simulink model "ofdm_txrx_supermimo"  (Simulink 7.1)
settings: Solver=VariableStepDiscrete  SolverMode=SingleTasking  StartTime=0.0  StopTime=simOnly_simLength  RelTol=1e-3
PostLoadFcn:
  | ofdm_rx_supermimo_init
InitFcn:
  | ofdm_rx_supermimo_init
StartFcn:
  | ofdm_rx_supermimo_init
values from neighbouring files (symbols this model uses):
  Action0_Config = 0* 31*2^18 + 0*2^24 + PHY_AUTORESPONSE_REQ_GOODHDR + PHY_AUTORESPONSE_REQ_GOODPKT + PHY_AUTORESPONSE_REQ_FLAGA    [ofdm_tx_supermimo_init.m]
  Action1_Config = 0* 62*2^18 + PHY_AUTORESPONSE_REQ_GOODHDR + PHY_AUTORESPONSE_REQ_GOODPKT    [ofdm_tx_supermimo_init.m]
  Action2_Config = 0    [ofdm_tx_supermimo_init.m]
  Action3_Config = 0    [ofdm_tx_supermimo_init.m]
  Action4_Config = 0    [ofdm_tx_supermimo_init.m]
  Action5_Config = 0    [ofdm_tx_supermimo_init.m]
  ActionID_AFTransmit = 31    [ofdm_tx_supermimo_init.m]
  ActionID_Disabled = 0    [ofdm_tx_supermimo_init.m]
  ActionID_SetFlagA = 62    [ofdm_tx_supermimo_init.m]
  ActionID_SetFlagB = 61    [ofdm_tx_supermimo_init.m]
  BER_RAM_init_size = 2048    [ofdm_tx_supermimo_init.m]
  CPLength = 16    [ofdm_tx_supermimo_init.m]
  longCorr_coef_i = [-4*real(LTS) 0]    [ofdm_rx_supermimo_init.m]
  longCorr_coef_q = [4*imag(LTS) 0]    [ofdm_rx_supermimo_init.m]
  Match0_Config = 0    [ofdm_tx_supermimo_init.m]
  Match1_Config = 0    [ofdm_tx_supermimo_init.m]
  Match2_Config = 0    [ofdm_tx_supermimo_init.m]
  Match3_Config = 0    [ofdm_tx_supermimo_init.m]
  Match4_Config = 0    [ofdm_tx_supermimo_init.m]
  Match5_Config = 0    [ofdm_tx_supermimo_init.m]
  Match6_Config = 0    [ofdm_tx_supermimo_init.m]
  Match7_Config = 0    [ofdm_tx_supermimo_init.m]
  max_CP_length = 16    [ofdm_tx_supermimo_init.m]
  max_num_baseRateSymbols = 31    [ofdm_tx_supermimo_init.m]
  max_num_subcarriers = 64    [ofdm_tx_supermimo_init.m]
  max_num_trainingSymbols = 15    [ofdm_tx_supermimo_init.m]
  max_numBytes = 16384    [ofdm_tx_supermimo_init.m]
  max_OFDM_symbols = 511    [ofdm_tx_supermimo_init.m]
  modConstellation_qam16 = .75*[1 3 -1 -3]./3    [ofdm_tx_supermimo_init.m]
  modConstellation_qam64 = 0.875*[3 1 5 7 -3 -1 -5 -7]./7    [ofdm_tx_supermimo_init.m]
  modConstellation_qpsk = [1 -1]./sqrt(2)    [ofdm_tx_supermimo_init.m]
  numBaseRateSymbols = ceil(numHeaderBytes / numBytes_BaseRateOFDMSymbol)    [ofdm_tx_supermimo_init.m]
  numSubcarriers = 64    [ofdm_tx_supermimo_init.m]
  numTrainingSymbols = 2    [ofdm_tx_supermimo_init.m]
  pilot_indicies = 7 + ( (64-21) * 2^8) + ( (64-7) * 2^16) + (21 * 2^24)    (= 356068103)    [ofdm_tx_supermimo_init.m]
  pilotValues = hex2dec('A57D') + (2^16 * hex2dec('5A82'))    [ofdm_tx_supermimo_init.m]
  pkt_crossCorr_thresh = 0.7    [ofdm_rx_supermimo_init.m]
  pkt_energy_thresh = 0    [ofdm_rx_supermimo_init.m]
  pktByteNums = numHeaderBytes +  				byteIndex_numPayloadBytes(1)*2^8 +  				byteIndex_numPayloadBytes(2)*2^16 +  				byteIndex_simpleDynModMasks * 2^24    [ofdm_rx_supermimo_init.m]
  PktDet_Delay = 58+32 +      Symbol_Timing_Offset*2^7 +      0*2^16 +      (117* 2^24)    (= 1962935642)    [ofdm_rx_supermimo_init.m]
  pktTiming_count_payloadStart = pktTiming_count_lsStart + pktTiming_ls_num*pktTiming_ls_length    [ofdm_rx_supermimo_init.m]
  preamble = preamble_scale*[shortsyms_10 longsyms_2]    [ofdm_tx_supermimo_init.m]
  preamble_ROM = preamble_I_ROM + preamble_Q_ROM*2^16    [ofdm_tx_supermimo_init.m]
  RAM_init_size = max_numBytes    (= 16384)    [ofdm_tx_supermimo_init.m]
  reg_AF_Tx_Blanking = (320+80) + ( (320+80+79)*2^16)    (= 31392144)    [ofdm_rx_supermimo_init.m]
  reg_AF_Tx_Scaling = 2944    [ofdm_rx_supermimo_init.m]
  reg_Interrupt_PktBuf_Control = reg_InterruptControl +  	1 * 2^16 +  	0 * 2^24    (= 65656)    [ofdm_rx_supermimo_init.m]
  reg_PilotCalcParams = (4 * 2^16) + (minPilotChanMag)    (= 262184.96)    [ofdm_rx_supermimo_init.m]
  regRx_chanEst_minMag = ((0.5)*2^15) + ((0.5 * 2^15)*2^16)    (= 1073758208)    [ofdm_rx_supermimo_init.m]
  regRx_coarseCalcCorrection = round((1.3e-3 - 1.2982e-3)*2^32)    [ofdm_rx_supermimo_init.m]
  regRx_pilotCalcCorrection = round(1.00115 * 2^31)    [ofdm_rx_supermimo_init.m]
  regRx_preCFOoptions = 0 * 2^0 +  	1 * 2^1 +  	0    (= 2)    [ofdm_rx_supermimo_init.m]
  rx_controlBits = 1 * 2  		 + 1 * 4  		 + 0 * 8  		 + 1 * 16 * rx_SISO_Mode  		 + 1 * 32  		 + 1 * 64  		 + 1 * 128  		 + 1 * 256  		 + 1 * 512  		 + 1 * 1024  		 + 1 * 4096  		 + 0 * 16384  		 + 0 * 32768  		 + 1 * 65536  		 + 0 * 131072  		 + 0 * 262144  		 + 1 * 524288  		 + 1 * 1048576 * tx_Alamouti_Mode  		 + 0 * 2097152           + 1 * 33554432  		 + 0 * 4194304  		 + 0 * 8388608  		 + 0 * 16777216  		 + 1 *  ...(+49 chars)    [ofdm_rx_supermimo_init.m]
  Rx_PktDet_LongCorr_Params = LongCorrCounterLoad + 0*2^8 + 0*2^16 + 255*2^24    (= 4278190325)    [ofdm_rx_supermimo_init.m]
  Rx_PktDet_LongCorrThresholds = 8000    [ofdm_rx_supermimo_init.m]
  rx_postEq_scaling = round(rxScaling*2^11) * (1 + 2^16)    [ofdm_rx_supermimo_init.m]
  RxDataScrambling_Seq = [40 198 78 63 82 173 102 245 48 111 172 115 147 230 216 93 72 65 62 2 205 242 122 90 128 83 105 97 73 10 5 252]    [ofdm_tx_supermimo_init.m]
  RxReg_FixedPktLen = 0    [ofdm_rx_supermimo_init.m]
  shortSymbol_time = shortSymbol_time(1:16).'    [ofdm_tx_supermimo_init.m]
  simOnly_numSamples = length(preamble)+( (numSubcarriers+CPLength)*(numTrainingSymbols + numBaseRateSymbols + numFullRateSymbols/2) )    [ofdm_tx_supermimo_init.m]
  train = [train train]    [ofdm_tx_supermimo_init.m]
  tx_controlBits = (antB_preambleShift * 2^4) + (2^1 * tx_Alamouti_Mode) +  	(2^0 * tx_SISO_Mode) + 4*0 + 8*1 + 0*256 + 512*0 + 1024*0 +  	0*hex2dec('1000') + 0*hex2dec('2000') + 0*hex2dec('4000') +  	1*hex2dec('8000') + 1*hex2dec('10000') + hex2dec('20000') +  	16*hex2dec('100000')    [ofdm_tx_supermimo_init.m]
  tx_delays = (50*2^0) + (0*2^8) + (30*2^12)    (= 122930)    [ofdm_tx_supermimo_init.m]
  tx_scaling = (3072) + (12288 * 2^16)    (= 805309440)    [ofdm_tx_supermimo_init.m]
  TxDataScrambling_Seq = [40 198 78 63 82 173 102 245 48 111 172 115 147 230 216 93 72 65 62 2 205 242 122 90 128 83 105 97 73 10 5 252]    [ofdm_tx_supermimo_init.m]
  txfilt_firpm = 0.95*txfilt_firpm./(max(txfilt_firpm))    [ofdm_tx_supermimo_init.m]
  txfilt_intfilt = [0 intfilt(4,4,.8)]    [ofdm_tx_supermimo_init.m]
  txReg_symbolCounts = (2^8 *numBaseRateSymbols ) + numTrainingSymbols    [ofdm_tx_supermimo_init.m]
  TxRx_FFTScaling = (3 * 2^0) +  	(2 * 2^2) +  	(1 * 2^4) +  	(1 * 2^6) +  	(1 * 2^8) +  	(0 * 2^10)    (= 347)    [ofdm_tx_supermimo_init.m]
undefined symbols (not defined in the file or in its folder): AntA, AntB, BaseRate, Down, gcbh, numBuffs, numBytes, Up, xlBlockLoadCallback, xlGetSimulinkPeriod, xlipgetpartsetting
libraries: xbsIndex_r4 x6035, simulink x4, dspsigops x4, commchan3 x2, dspsrcs4 x2, commrflib2 x2
(part 27 of 61)

== system ofdm_txrx_supermimo/OFDM Tx MIMO/Pre-Spin,  Filters & DACs/Filters/Interp Filt4 ==
  X : Inport  OutDataType=sfix(16)  OutScaling=2^0
  AddSub{1,3..4,7} (4 alike) : xbsIndex_r4/AddSub  mode=Addition  use_carryin=off  use_carryout=off  en=off  latency=0  precision=Full  arith_type=Unsigned  n_bits=16  bin_pt=14  quantization=Truncate  overflow=Wrap  dbl_ovrd=off  use_behavioral_HDL=off  pipelined=off  use_rpm=on  hw_selection=Fabric  block_type=addsub
      settings: block_version=10.1.3
  AddSub2 : xbsIndex_r4/AddSub  mode=Addition  use_carryin=off  use_carryout=off  en=off  latency=1  precision=Full  arith_type=Unsigned  n_bits=16  bin_pt=14  quantization=Truncate  overflow=Wrap  dbl_ovrd=off  use_behavioral_HDL=off  pipelined=off  use_rpm=on  hw_selection=Fabric  block_type=addsub
      settings: block_version=10.1.3
  AddSub5 : xbsIndex_r4/AddSub  mode=Addition  use_carryin=off  use_carryout=off  en=off  latency=1  precision=Full  arith_type=Unsigned  n_bits=16  bin_pt=14  quantization=Truncate  overflow=Wrap  dbl_ovrd=off  use_behavioral_HDL=off  pipelined=off  use_rpm=on  hw_selection=Fabric  block_type=addsub
      settings: block_version=10.1.3
  AddSub8 : xbsIndex_r4/AddSub  mode=Addition  use_carryin=off  use_carryout=off  en=off  latency=0  precision=User Defined  arith_type="Signed  (2's comp)"  n_bits=add_prec  bin_pt=add_bp  quantization=Truncate  overflow=Flag as error  dbl_ovrd=off  use_behavioral_HDL=off  pipelined=off  use_rpm=on  hw_selection=Fabric  block_type=addsub
      settings: block_version=10.1.3
  Counter : xbsIndex_r4/Counter  cnt_type=Free Running  cnt_to=Inf  operation=Up  start_count=1  cnt_by_val=1  arith_type=Unsigned  n_bits=2  bin_pt=0  load_pin=off  rst=off  en=off  explicit_period=on  period=data_samplePeriod / polyphaseBranches  dbl_ovrd=off  use_behavioral_HDL=off  use_rpm=off  implementation=Fabric  block_type=counter
  Delay{,1..6} (7 alike) : xbsIndex_r4/Delay  Orientation=down  en=off  latency=1  dbl_ovrd=off  reg_retiming=off  block_type=delay
  Mult{1..8} (8 alike) : xbsIndex_r4/Mult  precision=User Defined  arith_type="Signed  (2's comp)"  n_bits=mult_prec  bin_pt=mult_bp  quantization=Truncate  overflow=Flag as error  en=off  latency=multLatency  dbl_ovrd=off  use_behavioral_HDL=off  use_embedded=on  opt=Speed  optimum_pipeline=off  pipeline=on  use_rpm=on  placement_style=Triangular  block_type=mult
      settings: infoedit=Hardware notes: To use the internal pipeline stage of the dedicated multiplier you must select 'Pipeline for maximum performance'.  block_version=10.1.3
  ROM0 : xbsIndex_r4/ROM  depth=polyphaseBranches  initVector=h(1:subFilterLength)  distributed_mem=Distributed memory  rst=off  init_reg=0  en=off  latency=1  arith_type="Signed  (2's comp)"  n_bits=coeff_prec  bin_pt=coeff_bp  dbl_ovrd=off  optimize=Area  use_rpm=on  block_type=sprom
      settings: block_version=10.1.3
  ROM1 : xbsIndex_r4/ROM  depth=polyphaseBranches  initVector=h(1*subFilterLength+1:2*subFilterLength)  distributed_mem=Distributed memory  rst=off  init_reg=0  en=off  latency=1  arith_type="Signed  (2's comp)"  n_bits=coeff_prec  bin_pt=coeff_bp  dbl_ovrd=off  optimize=Area  use_rpm=on  block_type=sprom
      settings: block_version=10.1.3
  ROM2 : xbsIndex_r4/ROM  depth=polyphaseBranches  initVector=h(2*subFilterLength+1:3*subFilterLength)  distributed_mem=Distributed memory  rst=off  init_reg=0  en=off  latency=1  arith_type="Signed  (2's comp)"  n_bits=coeff_prec  bin_pt=coeff_bp  dbl_ovrd=off  optimize=Area  use_rpm=on  block_type=sprom
      settings: block_version=10.1.3
  ROM3 : xbsIndex_r4/ROM  depth=polyphaseBranches  initVector=h(3*subFilterLength+1:4*subFilterLength)  distributed_mem=Distributed memory  rst=off  init_reg=0  en=off  latency=1  arith_type="Signed  (2's comp)"  n_bits=coeff_prec  bin_pt=coeff_bp  dbl_ovrd=off  optimize=Area  use_rpm=on  block_type=sprom
      settings: block_version=10.1.3
  ROM4 : xbsIndex_r4/ROM  depth=polyphaseBranches  initVector=h(4*subFilterLength+1:5*subFilterLength)  distributed_mem=Distributed memory  rst=off  init_reg=0  en=off  latency=1  arith_type="Signed  (2's comp)"  n_bits=coeff_prec  bin_pt=coeff_bp  dbl_ovrd=off  optimize=Area  use_rpm=on  block_type=sprom
      settings: block_version=10.1.3
  ROM5 : xbsIndex_r4/ROM  depth=polyphaseBranches  initVector=h(5*subFilterLength+1:6*subFilterLength)  distributed_mem=Distributed memory  rst=off  init_reg=0  en=off  latency=1  arith_type="Signed  (2's comp)"  n_bits=coeff_prec  bin_pt=coeff_bp  dbl_ovrd=off  optimize=Area  use_rpm=on  block_type=sprom
      settings: block_version=10.1.3
  ROM6 : xbsIndex_r4/ROM  depth=polyphaseBranches  initVector=h(6*subFilterLength+1:7*subFilterLength)  distributed_mem=Distributed memory  rst=off  init_reg=0  en=off  latency=1  arith_type="Signed  (2's comp)"  n_bits=coeff_prec  bin_pt=coeff_bp  dbl_ovrd=off  optimize=Area  use_rpm=on  block_type=sprom
      settings: block_version=10.1.3
  ROM7 : xbsIndex_r4/ROM  depth=polyphaseBranches  initVector=h(7*subFilterLength+1:8*subFilterLength)  distributed_mem=Distributed memory  rst=off  init_reg=0  en=off  latency=1  arith_type="Signed  (2's comp)"  n_bits=coeff_prec  bin_pt=coeff_bp  dbl_ovrd=off  optimize=Area  use_rpm=on  block_type=sprom
      settings: block_version=10.1.3
  Up Sample{8..15} (8 alike) : xbsIndex_r4/Up Sample  sample_ratio=polyphaseBranches  copy_samples=on  dbl_ovrd=off  block_type=usamp
      settings: infoedit="Up samples input data.  Inserted values  can be zeros or copies of the most recent input sample.<P><P>Hardware notes: No hardware is needed if inserted values are copies of the input sample; otherwise, a mux and single bit flip-flop are used."  block_version=10.1.3
  Y : Outport  OutDataType=sfix(16)  OutScaling=2^0
  wires:
  AddSub8/1 -> Y/1
  Up Sample15/1 -> Mult5/1
  Up Sample14/1 -> Mult6/1
  Up Sample13/1 -> Mult4/1
  Up Sample12/1 -> Mult7/1
  Up Sample11/1 -> Mult2/1
  Up Sample10/1 -> Mult3/1
  Up Sample9/1 -> Mult1/1
  Up Sample8/1 -> Mult8/1
  AddSub5/1 -> AddSub8/2
  AddSub2/1 -> AddSub8/1
  Mult5/1 -> AddSub4/2
  Mult6/1 -> AddSub4/1
  Mult4/1 -> AddSub3/2
  Mult7/1 -> AddSub3/1
  AddSub4/1 -> AddSub5/2
  AddSub3/1 -> AddSub5/1
  AddSub1/1 -> AddSub2/2
  AddSub7/1 -> AddSub2/1
  Mult2/1 -> AddSub1/2
  Mult3/1 -> AddSub1/1
  Mult1/1 -> AddSub7/2
  Mult8/1 -> AddSub7/1
  Counter/1 -> ROM7/1, ROM6/1, ROM5/1, ROM4/1, ROM3/1, ROM2/1, ROM1/1, ROM0/1
  ROM7/1 -> Mult5/2
  ROM6/1 -> Mult6/2
  ROM5/1 -> Mult4/2
  ROM4/1 -> Mult7/2
  ROM3/1 -> Mult2/2
  ROM2/1 -> Mult3/2
  ROM1/1 -> Mult1/2
  ROM0/1 -> Mult8/2
  X/1 -> Delay/1, Up Sample8/1
  Delay6/1 -> Up Sample15/1
  Delay5/1 -> Delay6/1, Up Sample14/1
  Delay4/1 -> Delay5/1, Up Sample13/1
  Delay3/1 -> Delay4/1, Up Sample12/1
  Delay2/1 -> Delay3/1, Up Sample11/1
  Delay1/1 -> Delay2/1, Up Sample10/1
  Delay/1 -> Delay1/1, Up Sample9/1

== system ofdm_txrx_supermimo/OFDM Tx MIMO/Pre-Spin,  Filters & DACs/Filters/Interp Filt5 ==
  X : Inport  OutDataType=sfix(16)  OutScaling=2^0
  AddSub{1,3..4,7} (4 alike) : xbsIndex_r4/AddSub  mode=Addition  use_carryin=off  use_carryout=off  en=off  latency=0  precision=Full  arith_type=Unsigned  n_bits=16  bin_pt=14  quantization=Truncate  overflow=Wrap  dbl_ovrd=off  use_behavioral_HDL=off  pipelined=off  use_rpm=on  hw_selection=Fabric  block_type=addsub
      settings: block_version=10.1.3
  AddSub2 : xbsIndex_r4/AddSub  mode=Addition  use_carryin=off  use_carryout=off  en=off  latency=1  precision=Full  arith_type=Unsigned  n_bits=16  bin_pt=14  quantization=Truncate  overflow=Wrap  dbl_ovrd=off  use_behavioral_HDL=off  pipelined=off  use_rpm=on  hw_selection=Fabric  block_type=addsub
      settings: block_version=10.1.3
  AddSub5 : xbsIndex_r4/AddSub  mode=Addition  use_carryin=off  use_carryout=off  en=off  latency=1  precision=Full  arith_type=Unsigned  n_bits=16  bin_pt=14  quantization=Truncate  overflow=Wrap  dbl_ovrd=off  use_behavioral_HDL=off  pipelined=off  use_rpm=on  hw_selection=Fabric  block_type=addsub
      settings: block_version=10.1.3
  AddSub8 : xbsIndex_r4/AddSub  mode=Addition  use_carryin=off  use_carryout=off  en=off  latency=0  precision=User Defined  arith_type="Signed  (2's comp)"  n_bits=add_prec  bin_pt=add_bp  quantization=Truncate  overflow=Flag as error  dbl_ovrd=off  use_behavioral_HDL=off  pipelined=off  use_rpm=on  hw_selection=Fabric  block_type=addsub
      settings: block_version=10.1.3
  Counter : xbsIndex_r4/Counter  cnt_type=Free Running  cnt_to=Inf  operation=Up  start_count=1  cnt_by_val=1  arith_type=Unsigned  n_bits=2  bin_pt=0  load_pin=off  rst=off  en=off  explicit_period=on  period=data_samplePeriod / polyphaseBranches  dbl_ovrd=off  use_behavioral_HDL=off  use_rpm=off  implementation=Fabric  block_type=counter
  Delay{,1..6} (7 alike) : xbsIndex_r4/Delay  Orientation=down  en=off  latency=1  dbl_ovrd=off  reg_retiming=off  block_type=delay
  Mult{1..8} (8 alike) : xbsIndex_r4/Mult  precision=User Defined  arith_type="Signed  (2's comp)"  n_bits=mult_prec  bin_pt=mult_bp  quantization=Truncate  overflow=Flag as error  en=off  latency=multLatency  dbl_ovrd=off  use_behavioral_HDL=off  use_embedded=on  opt=Speed  optimum_pipeline=off  pipeline=on  use_rpm=on  placement_style=Triangular  block_type=mult
      settings: infoedit=Hardware notes: To use the internal pipeline stage of the dedicated multiplier you must select 'Pipeline for maximum performance'.  block_version=10.1.3
  ROM0 : xbsIndex_r4/ROM  depth=polyphaseBranches  initVector=h(1:subFilterLength)  distributed_mem=Distributed memory  rst=off  init_reg=0  en=off  latency=1  arith_type="Signed  (2's comp)"  n_bits=coeff_prec  bin_pt=coeff_bp  dbl_ovrd=off  optimize=Area  use_rpm=on  block_type=sprom
      settings: block_version=10.1.3
  ROM1 : xbsIndex_r4/ROM  depth=polyphaseBranches  initVector=h(1*subFilterLength+1:2*subFilterLength)  distributed_mem=Distributed memory  rst=off  init_reg=0  en=off  latency=1  arith_type="Signed  (2's comp)"  n_bits=coeff_prec  bin_pt=coeff_bp  dbl_ovrd=off  optimize=Area  use_rpm=on  block_type=sprom
      settings: block_version=10.1.3
  ROM2 : xbsIndex_r4/ROM  depth=polyphaseBranches  initVector=h(2*subFilterLength+1:3*subFilterLength)  distributed_mem=Distributed memory  rst=off  init_reg=0  en=off  latency=1  arith_type="Signed  (2's comp)"  n_bits=coeff_prec  bin_pt=coeff_bp  dbl_ovrd=off  optimize=Area  use_rpm=on  block_type=sprom
      settings: block_version=10.1.3
  ROM3 : xbsIndex_r4/ROM  depth=polyphaseBranches  initVector=h(3*subFilterLength+1:4*subFilterLength)  distributed_mem=Distributed memory  rst=off  init_reg=0  en=off  latency=1  arith_type="Signed  (2's comp)"  n_bits=coeff_prec  bin_pt=coeff_bp  dbl_ovrd=off  optimize=Area  use_rpm=on  block_type=sprom
      settings: block_version=10.1.3
  ROM4 : xbsIndex_r4/ROM  depth=polyphaseBranches  initVector=h(4*subFilterLength+1:5*subFilterLength)  distributed_mem=Distributed memory  rst=off  init_reg=0  en=off  latency=1  arith_type="Signed  (2's comp)"  n_bits=coeff_prec  bin_pt=coeff_bp  dbl_ovrd=off  optimize=Area  use_rpm=on  block_type=sprom
      settings: block_version=10.1.3
  ROM5 : xbsIndex_r4/ROM  depth=polyphaseBranches  initVector=h(5*subFilterLength+1:6*subFilterLength)  distributed_mem=Distributed memory  rst=off  init_reg=0  en=off  latency=1  arith_type="Signed  (2's comp)"  n_bits=coeff_prec  bin_pt=coeff_bp  dbl_ovrd=off  optimize=Area  use_rpm=on  block_type=sprom
      settings: block_version=10.1.3
  ROM6 : xbsIndex_r4/ROM  depth=polyphaseBranches  initVector=h(6*subFilterLength+1:7*subFilterLength)  distributed_mem=Distributed memory  rst=off  init_reg=0  en=off  latency=1  arith_type="Signed  (2's comp)"  n_bits=coeff_prec  bin_pt=coeff_bp  dbl_ovrd=off  optimize=Area  use_rpm=on  block_type=sprom
      settings: block_version=10.1.3
  ROM7 : xbsIndex_r4/ROM  depth=polyphaseBranches  initVector=h(7*subFilterLength+1:8*subFilterLength)  distributed_mem=Distributed memory  rst=off  init_reg=0  en=off  latency=1  arith_type="Signed  (2's comp)"  n_bits=coeff_prec  bin_pt=coeff_bp  dbl_ovrd=off  optimize=Area  use_rpm=on  block_type=sprom
      settings: block_version=10.1.3
  Up Sample{8..15} (8 alike) : xbsIndex_r4/Up Sample  sample_ratio=polyphaseBranches  copy_samples=on  dbl_ovrd=off  block_type=usamp
      settings: infoedit="Up samples input data.  Inserted values  can be zeros or copies of the most recent input sample.<P><P>Hardware notes: No hardware is needed if inserted values are copies of the input sample; otherwise, a mux and single bit flip-flop are used."  block_version=10.1.3
  Y : Outport  OutDataType=sfix(16)  OutScaling=2^0
  wires:
  Delay/1 -> Up Sample9/1, Delay1/1
  Delay1/1 -> Up Sample10/1, Delay2/1
  Delay2/1 -> Up Sample11/1, Delay3/1
  Delay3/1 -> Up Sample12/1, Delay4/1
  Delay4/1 -> Up Sample13/1, Delay5/1
  Delay5/1 -> Up Sample14/1, Delay6/1
  Delay6/1 -> Up Sample15/1
  X/1 -> Up Sample8/1, Delay/1
  ROM0/1 -> Mult8/2
  ROM1/1 -> Mult1/2
  ROM2/1 -> Mult3/2
  ROM3/1 -> Mult2/2
  ROM4/1 -> Mult7/2
  ROM5/1 -> Mult4/2
  ROM6/1 -> Mult6/2
  ROM7/1 -> Mult5/2
  Counter/1 -> ROM0/1, ROM1/1, ROM2/1, ROM3/1, ROM4/1, ROM5/1, ROM6/1, ROM7/1
  Mult8/1 -> AddSub7/1
  Mult1/1 -> AddSub7/2
  Mult3/1 -> AddSub1/1
  Mult2/1 -> AddSub1/2
  AddSub7/1 -> AddSub2/1
  AddSub1/1 -> AddSub2/2
  AddSub3/1 -> AddSub5/1
  AddSub4/1 -> AddSub5/2
  Mult7/1 -> AddSub3/1
  Mult4/1 -> AddSub3/2
  Mult6/1 -> AddSub4/1
  Mult5/1 -> AddSub4/2
  AddSub2/1 -> AddSub8/1
  AddSub5/1 -> AddSub8/2
  Up Sample8/1 -> Mult8/1
  Up Sample9/1 -> Mult1/1
  Up Sample10/1 -> Mult3/1
  Up Sample11/1 -> Mult2/1
  Up Sample12/1 -> Mult7/1
  Up Sample13/1 -> Mult4/1
  Up Sample14/1 -> Mult6/1
  Up Sample15/1 -> Mult5/1
  AddSub8/1 -> Y/1

== system ofdm_txrx_supermimo/OFDM Tx MIMO/Training_Data/Ant Selector/Oscillator ==
  rst : Inport  OutDataType=sfix(16)  OutScaling=2^0
  en : Inport  Port=2  OutDataType=sfix(16)  OutScaling=2^0
  Assert : xbsIndex_r4/Assert  Orientation=left  assert_type=on  type_source=Explicitly  arith_type=Boolean  n_bits=16  bin_pt=14  assert_rate=on  rate_source=Explicitly  period=2  output_port=on  block_type=assert
      settings: infoedit=Asserts a user-defined sample rate and/or type on a signal.<P><P>Hardware notes: In hardware this block costs nothing.  block_version=VER_STRING_GOES_HERE
  Inverter1 : xbsIndex_r4/Inverter  Orientation=left  en=off  latency=0  dbl_ovrd=off  block_type=inv
      settings: block_version=VER_STRING_GOES_HERE
  Register : xbsIndex_r4/Register  init=0  rst=on  en=on  dbl_ovrd=off  block_type=register
      settings: block_version=VER_STRING_GOES_HERE
  Q : Outport  OutDataType=sfix(16)  OutScaling=2^0
  wires:
  en/1 -> Register/3
  Inverter1/1 -> Register/1
  Assert/1 -> Inverter1/1
  Register/1 -> Assert/1, Q/1
  rst/1 -> Register/2

== system ofdm_txrx_supermimo/OFDM Tx MIMO/Training_Data/Ant Selector/negedge ==
  D : Inport  OutDataType=sfix(16)  OutScaling=2^0
  Delay : xbsIndex_r4/Delay  en=off  latency=1  dbl_ovrd=off  reg_retiming=on  block_type=delay
      settings: infoedit=Hardware notes: A delay line is a chain, each link of which is an SRL16 followed by a flip-flop. If register retiming is enabled, the delay line is a chain of flip-flops.  block_version=VER_STRING_GOES_HERE
  Inverter1 : xbsIndex_r4/Inverter  en=off  latency=0  dbl_ovrd=off  block_type=inv
      settings: block_version=VER_STRING_GOES_HERE
  Logical3 : xbsIndex_r4/Logical  logical_function=AND  inputs=2  en=off  latency=0  precision=Full  arith_type=Unsigned  n_bits=16  bin_pt=0  align_bp=on  dbl_ovrd=off  block_type=logical
      settings: block_version=VER_STRING_GOES_HERE
  Q : Outport  OutDataType=sfix(16)  OutScaling=2^0
  wires:
  Inverter1/1 -> Logical3/1
  D/1 -> Inverter1/1, Delay/1
  Delay/1 -> Logical3/2
  Logical3/1 -> Q/1

== system ofdm_txrx_supermimo/OFDM Tx MIMO/Training_Data/FlexibleMod/PktBuffer_CRC1 ==
  Rst : Inport  OutDataType=sfix(16)  OutScaling=2^0
  DataOut_Addr Vin : Inport  Port=2  OutDataType=sfix(16)  OutScaling=2^0
  DataOut_Addr : Inport  Port=3  OutDataType=sfix(16)  OutScaling=2^0
  Assert : xbsIndex_r4/Assert  Orientation=left  assert_type=off  type_source=Explicitly  arith_type="Signed  (2's comp)"  n_bits=16  bin_pt=14  assert_rate=on  rate_source=Explicitly  period=2  output_port=on  block_type=assert
      settings: infoedit=Asserts a user-defined sample rate and/or type on a signal.<P><P>Hardware notes: In hardware this block costs nothing.  block_version=8.2.01
  Byte Selector : Subsystem
  CRC Length Calc1 : Subsystem
  CRC16 Calc : Subsystem
  CRC32 Calc : Subsystem
  Gateway Out1 : xbsIndex_r4/Gateway Out  hdl_port=off  timing_constraint=None  locs_specified=off  LOCs={}  block_type=gatewayout
      settings: infoedit="Gateway out block.  Converts Xilinx fixed point inputs into ouputs of type Simulink integer, double, or fixed point.<P><P>Hardware notes:  In hardware these blocks become top level output ports or are discarded, depending on how they are configured."  block_version=10.1.3
  Gateway Out4 : xbsIndex_r4/Gateway Out  hdl_port=off  timing_constraint=None  locs_specified=off  LOCs={}  block_type=gatewayout
      settings: infoedit="Gateway out block.  Converts Xilinx fixed point inputs into ouputs of type Simulink integer, double, or fixed point.<P><P>Hardware notes:  In hardware these blocks become top level output ports or are discarded, depending on how they are configured."  block_version=10.1.3
  Header Decoding1 : Subsystem
  Logical8 : xbsIndex_r4/Logical  logical_function=OR  inputs=2  en=off  latency=0  precision=Full  arith_type=Unsigned  n_bits=8  bin_pt=2  align_bp=on  dbl_ovrd=off  block_type=logical
      settings: xl_area=[0, 0, 0, 0, 0, 0, 0]  block_version=VER_STRING_GOES_HERE
  Mux : xbsIndex_r4/Mux  inputs=2  en=off  latency=0  precision=Full  arith_type="Signed  (2's comp)"  n_bits=8  bin_pt=2  quantization=Truncate  overflow=Wrap  dbl_ovrd=off  block_type=mux
      settings: xl_area=[0, 0, 0, 0, 0, 0, 0]  block_version=VER_STRING_GOES_HERE
  Packet Buffer : Subsystem
  Register{1,3..6} (5 alike) : xbsIndex_r4/Register  init=0  rst=off  en=off  dbl_ovrd=off  block_type=register
      settings: xl_area=[2 4 0 0 0 0 0]  block_version=VER_STRING_GOES_HERE
  Register2 : xbsIndex_r4/Register  Orientation=up  init=0  rst=off  en=off  dbl_ovrd=off  block_type=register
      settings: xl_area=[2 4 0 0 0 0 0]  block_version=VER_STRING_GOES_HERE
  Scrambling : Subsystem
  Tx Bytes : Scope  NumInputPorts=2
      settings: TimeRange=7000  YMin=0~0  YMax=3~4e+009
  NumBytes : Outport  OutDataType=sfix(16)  OutScaling=2^0
  FullRateMasks : Outport  Port=2  OutDataType=sfix(16)  OutScaling=2^0
  Dout : Outport  Port=3  OutDataType=sfix(16)  OutScaling=2^0
  wires:
  Header Decoding1/1(numBytes) -> NumBytes/1, CRC Length Calc1/1(NumBytes)
  Header Decoding1/2(modMask) -> FullRateMasks/1
  DataOut_Addr/1 -> Packet Buffer/1(TxByteAddr), Register2/1, Register4/1
  Scrambling/1(Dout) -> Dout/1
  Mux/1 -> Scrambling/1(Din), Assert/1, Gateway Out4/1
  Packet Buffer/1(ByteOut) -> Mux/2, Header Decoding1/2(TxByte)
  DataOut_Addr Vin/1 -> Register3/1
  Rst/1 -> Register1/1
  CRC Length Calc1/3(CRC_ByteSel) -> Byte Selector/2(ByteSel)
  Byte Selector/1(1 Byte Out) -> Mux/3
  CRC Length Calc1/4(CRC32_Capt) -> CRC32 Calc/4(Capt)
  Assert/1 -> CRC16 Calc/1(ByteIn), CRC32 Calc/1(ByteIn)
  Register3/1 -> Header Decoding1/3(TxByteAddrVin), CRC Length Calc1/3(Vin), CRC32 Calc/2(Vin), CRC16 Calc/2(Vin)
  Register1/1 -> CRC32 Calc/3(Rst), Header Decoding1/4(Rst), CRC16 Calc/3(Rst)
  Register2/1 -> Header Decoding1/1(TxByteAddr), Register6/1, Scrambling/2(Addr)
  CRC32 Calc/1(32-bit CRC Capt) -> Byte Selector/3(CRC32)
  CRC Length Calc1/5(CRC16_Capt) -> CRC16 Calc/4(Capt)
  CRC Length Calc1/1(CRC32_En) -> Logical8/1, Byte Selector/1(CRC32_en)
  CRC Length Calc1/2(CRC16_En) -> Logical8/2
  Logical8/1 -> Mux/1
  CRC16 Calc/1(16-bit CRC Capt) -> Byte Selector/4(CRC16)
  Gateway Out4/1 -> Tx Bytes/1
  Gateway Out1/1 -> Tx Bytes/2
  Register4/1 -> Register5/1
  Register5/1 -> Gateway Out1/1
  Register6/1 -> CRC Length Calc1/2(ByteAddr)

== system ofdm_txrx_supermimo/OFDM Tx MIMO/Training_Data/FlexibleMod/QAM ==
  note: Mod-masks are interpreted as literal bits/symbol value. Only
      | BPSK/QPSK/16QAM/64QAM are implemented (1/2/4/6 b/sym),
      | but other schemes could be added in this block to fill the unused
      | mod mask values.
  Mod Bits : Inport  OutDataType=sfix(16)  OutScaling=2^0
  Mod Sel : Inport  Port=2  OutDataType=sfix(16)  OutScaling=2^0
  16QAM Mapper : xbsIndex_r4/ROM  depth=max(size(modConstellation_qam16))  initVector=modConstellation_qam16  distributed_mem=Distributed memory  rst=off  init_reg=0  en=off  latency=1  arith_type="Signed  (2's comp)"  n_bits=modConstellation_prec  bin_pt=modConstellation_bp  dbl_ovrd=off  optimize=Area  use_rpm=off  block_type=sprom
      settings: xl_area=[4 8 0 8 0 0 0]  block_version=VER_STRING_GOES_HERE
  16QAM Mapper1 : xbsIndex_r4/ROM  depth=max(size(modConstellation_qam16))  initVector=modConstellation_qam16  distributed_mem=Distributed memory  rst=off  init_reg=0  en=off  latency=1  arith_type="Signed  (2's comp)"  n_bits=modConstellation_prec  bin_pt=modConstellation_bp  dbl_ovrd=off  optimize=Area  use_rpm=off  block_type=sprom
      settings: xl_area=[4 8 0 8 0 0 0]  block_version=VER_STRING_GOES_HERE
  1MSB : xbsIndex_r4/Slice  nbits=1  boolean_output=off  mode=Upper Bit Location + Width  bit1=0  base1=MSB of Input  bit0=0  base0=LSB of Input  dbl_ovrd=off  block_type=slice
      settings: infoedit="Extracts a given range of bits from each input sample and presents it at the output.  The output type is ordinarily unsigned with binary point at zero, but can be Boolean when the slice is one bit wide.<P><P>Hardware notes: In hardware this block costs nothing."  block_version=VER_STRING_GOES_HERE
  1MSB-1 : xbsIndex_r4/Slice  nbits=1  boolean_output=off  mode=Upper Bit Location + Width  bit1=-1  base1=MSB of Input  bit0=0  base0=LSB of Input  dbl_ovrd=off  block_type=slice
      settings: infoedit="Extracts a given range of bits from each input sample and presents it at the output.  The output type is ordinarily unsigned with binary point at zero, but can be Boolean when the slice is one bit wide.<P><P>Hardware notes: In hardware this block costs nothing."  block_version=VER_STRING_GOES_HERE
  2MSB : xbsIndex_r4/Slice  nbits=2  boolean_output=off  mode=Upper Bit Location + Width  bit1=0  base1=MSB of Input  bit0=0  base0=LSB of Input  dbl_ovrd=off  block_type=slice
      settings: infoedit="Extracts a given range of bits from each input sample and presents it at the output.  The output type is ordinarily unsigned with binary point at zero, but can be Boolean when the slice is one bit wide.<P><P>Hardware notes: In hardware this block costs nothing."  block_version=VER_STRING_GOES_HERE
  2MSB-2 : xbsIndex_r4/Slice  nbits=2  boolean_output=off  mode=Upper Bit Location + Width  bit1=-2  base1=MSB of Input  bit0=0  base0=LSB of Input  dbl_ovrd=off  block_type=slice
      settings: infoedit="Extracts a given range of bits from each input sample and presents it at the output.  The output type is ordinarily unsigned with binary point at zero, but can be Boolean when the slice is one bit wide.<P><P>Hardware notes: In hardware this block costs nothing."  block_version=VER_STRING_GOES_HERE
  3LSB : xbsIndex_r4/Slice  nbits=3  boolean_output=off  mode=Lower Bit Location + Width  bit1=0  base1=MSB of Input  bit0=0  base0=LSB of Input  dbl_ovrd=off  block_type=slice
      settings: infoedit="Extracts a given range of bits from each input sample and presents it at the output.  The output type is ordinarily unsigned with binary point at zero, but can be Boolean when the slice is one bit wide.<P><P>Hardware notes: In hardware this block costs nothing."  block_version=VER_STRING_GOES_HERE
  3MS-3 : xbsIndex_r4/Slice  nbits=3  boolean_output=off  mode=Upper Bit Location + Width  bit1=-3  base1=MSB of Input  bit0=0  base0=LSB of Input  dbl_ovrd=off  block_type=slice
      settings: infoedit="Extracts a given range of bits from each input sample and presents it at the output.  The output type is ordinarily unsigned with binary point at zero, but can be Boolean when the slice is one bit wide.<P><P>Hardware notes: In hardware this block costs nothing."  block_version=VER_STRING_GOES_HERE
  3MSB : xbsIndex_r4/Slice  nbits=3  boolean_output=off  mode=Upper Bit Location + Width  bit1=0  base1=MSB of Input  bit0=0  base0=LSB of Input  dbl_ovrd=off  block_type=slice
      settings: infoedit="Extracts a given range of bits from each input sample and presents it at the output.  The output type is ordinarily unsigned with binary point at zero, but can be Boolean when the slice is one bit wide.<P><P>Hardware notes: In hardware this block costs nothing."  block_version=VER_STRING_GOES_HERE
  64QAM Mapper : xbsIndex_r4/ROM  depth=max(size(modConstellation_qam64))  initVector=modConstellation_qam64  distributed_mem=Distributed memory  rst=off  init_reg=0  en=off  latency=1  arith_type="Signed  (2's comp)"  n_bits=modConstellation_prec  bin_pt=modConstellation_bp  dbl_ovrd=off  optimize=Area  use_rpm=off  block_type=sprom
      settings: xl_area=[4 8 0 8 0 0 0]  block_version=VER_STRING_GOES_HERE
  64QAM Mapper1 : xbsIndex_r4/ROM  depth=max(size(modConstellation_qam64))  initVector=modConstellation_qam64  distributed_mem=Distributed memory  rst=off  init_reg=0  en=off  latency=1  arith_type="Signed  (2's comp)"  n_bits=modConstellation_prec  bin_pt=modConstellation_bp  dbl_ovrd=off  optimize=Area  use_rpm=off  block_type=sprom
      settings: xl_area=[4 8 0 8 0 0 0]  block_version=VER_STRING_GOES_HERE
  Constant7 : xbsIndex_r4/Constant  arith_type=Signed (2's comp)  const=0  n_bits=1  bin_pt=0  explicit_period=off  period=1  equ=P=C  opselect=C  inp2=PCIN>>17  opr=+  inp1=P  carry=CIN  dbl_ovrd=off  block_type=constant
      settings: block_version=VER_STRING_GOES_HERE
  Delay : xbsIndex_r4/Delay  en=off  latency=1  dbl_ovrd=off  reg_retiming=off  block_type=delay
      settings: infoedit=Hardware notes: A delay line is a chain, each link of which is an SRL16 followed by a flip-flop. If register retiming is enabled, the delay line is a chain of flip-flops.  xl_area=[2 4 0 0 0 0 0]  block_version=VER_STRING_GOES_HERE
  Mux1 : xbsIndex_r4/Mux  inputs=7  en=off  latency=0  precision=Full  arith_type="Signed  (2's comp)"  n_bits=8  bin_pt=2  quantization=Truncate  overflow=Wrap  dbl_ovrd=off  block_type=mux
      settings: xl_area=[36 0 0 72 0 0 0]  block_version=VER_STRING_GOES_HERE
  Mux2 : xbsIndex_r4/Mux  inputs=7  en=off  latency=0  precision=Full  arith_type="Signed  (2's comp)"  n_bits=8  bin_pt=2  quantization=Truncate  overflow=Wrap  dbl_ovrd=off  block_type=mux
      settings: xl_area=[36 0 0 72 0 0 0]  block_version=VER_STRING_GOES_HERE
  QPSK Map : Subsystem
  QPSK Map1 : Subsystem
  I : Outport  OutDataType=sfix(16)  OutScaling=2^0
  Q : Outport  Port=2  OutDataType=sfix(16)  OutScaling=2^0
  wires:
  1MSB-1/1 -> QPSK Map1/1(Bit)
  1MSB/1 -> QPSK Map/1(Bit)
  2MSB-2/1 -> 16QAM Mapper1/1
  2MSB/1 -> 16QAM Mapper/1
  Mod Bits/1 -> 1MSB/1, 2MSB/1, 3MSB/1, 2MSB-2/1, 3MS-3/1, 1MSB-1/1
  Mux2/1 -> I/1
  Mux1/1 -> Q/1
  3MS-3/1 -> 64QAM Mapper1/1
  3MSB/1 -> 64QAM Mapper/1
  QPSK Map1/1(Sym) -> Mux1/4
  16QAM Mapper1/1 -> Mux1/6
  64QAM Mapper1/1 -> Mux1/8
  Mod Sel/1 -> 3LSB/1
  Delay/1 -> Mux2/1, Mux1/1
  Constant7/1 -> Mux2/2, Mux2/5, Mux2/7, Mux1/3, Mux1/5, Mux1/7, Mux1/2
  QPSK Map/1(Sym) -> Mux2/3, Mux2/4
  16QAM Mapper/1 -> Mux2/6
  64QAM Mapper/1 -> Mux2/8
  3LSB/1 -> Delay/1

== system ofdm_txrx_supermimo/OFDM Tx MIMO/Training_Data/FlexibleMod/S-R Latch1 ==
  R : Inport  OutDataType=sfix(16)  OutScaling=2^0
  S : Inport  Port=2  OutDataType=sfix(16)  OutScaling=2^0
  Constant3 : xbsIndex_r4/Constant  arith_type=Boolean  const=1  n_bits=1  bin_pt=0  explicit_period=off  period=1  equ=P=C  opselect=C  inp2=PCIN>>17  opr=+  inp1=P  carry=CIN  dbl_ovrd=off  block_type=constant
      settings: block_version=VER_STRING_GOES_HERE
  Register : xbsIndex_r4/Register  init=0  rst=on  en=on  dbl_ovrd=off  block_type=register
      settings: xl_area=[0, 0, 0, 0, 0, 0, 0]  block_version=VER_STRING_GOES_HERE
  Q : Outport  OutDataType=sfix(16)  OutScaling=2^0
  wires:
  Constant3/1 -> Register/1
  R/1 -> Register/2
  Register/1 -> Q/1
  S/1 -> Register/3

== system ofdm_txrx_supermimo/OFDM Tx MIMO/Training_Data/FlexibleMod/SR Latch ==
  R : Inport  OutDataType=sfix(16)  OutScaling=2^0
  S : Inport  Port=2  OutDataType=sfix(16)  OutScaling=2^0
  Constant2 : xbsIndex_r4/Constant  arith_type=Boolean  const=1  n_bits=1  bin_pt=0  explicit_period=off  period=1  equ=P=C  opselect=C  inp2=PCIN>>17  opr=+  inp1=P  carry=CIN  dbl_ovrd=off  block_type=constant
      settings: block_version=VER_STRING_GOES_HERE
  Convert : xbsIndex_r4/Convert  arith_type=Boolean  n_bits=16  bin_pt=14  quantization=Truncate  overflow=Wrap  latency=0  dbl_ovrd=off  pipeline=off  block_type=convert
      settings: block_version=8.2.01
  Convert1 : xbsIndex_r4/Convert  arith_type=Boolean  n_bits=16  bin_pt=14  quantization=Truncate  overflow=Wrap  latency=0  dbl_ovrd=off  pipeline=off  block_type=convert
      settings: block_version=8.2.01
  crc_accum2 : xbsIndex_r4/Register  init=0  rst=on  en=on  dbl_ovrd=off  block_type=register
      settings: xl_area=[0, 0, 0, 0, 0, 0, 0]  block_version=VER_STRING_GOES_HERE
  Q : Outport  OutDataType=sfix(16)  OutScaling=2^0
  wires:
  Constant2/1 -> crc_accum2/1
  R/1 -> Convert/1
  S/1 -> Convert1/1
  crc_accum2/1 -> Q/1
  Convert/1 -> crc_accum2/2
  Convert1/1 -> crc_accum2/3

== system ofdm_txrx_supermimo/OFDM Tx MIMO/Training_Data/FlexibleMod/control ==
  note: This input, sub sync, gives the user
      |  the ability to reset the subcarrier
      | counter so that the user can
      | control what subcarrier the system starts
      | putting data on.
  note: This input, ready, is a bit that
      | is asserted when the user
      | is ready for the system to run,
      | i.e. the user has loaded information
      |  into the memory and is ready to
      | handle the output.
  note: This input, length, is the length
      | of the packet loaded in memory.
  note: This output puts out a pulse when the entire
      | system is done taking the bytes from the memory.
  note: This output, mod, is the desired modulation
      | type for a given group of bits. The values of this
      | output is designated by the values in
      |  subcarrier_QAM_Values in the init file
  note: This ouput, index, provides the index for
      | the location in the two registersos  so that the registers
      | can know when to load and the slicer can know
      | which bits to slice off of the 16-bit output of data_buffer
  note: This subsystem acts as the control unit for the rest of the system.
      | It uses a state system to control the system with three states, on, resetting, and off.
      | The number of subcarriers should be a power of two.
  FullRateMasks : Inport  OutDataType=sfix(16)  OutScaling=2^0
  length : Inport  Port=2  OutDataType=sfix(16)  OutScaling=2^0
  ready : Inport  Port=3  OutDataType=sfix(16)  OutScaling=2^0
  ifft_index : Inport  Port=4  OutDataType=sfix(16)  OutScaling=2^0
  BaseRate_En : Inport  Port=5  OutDataType=sfix(16)  OutScaling=2^0
  valid : Inport  Port=6  OutDataType=sfix(16)  OutScaling=2^0
  ant_sel : Inport  Port=7  OutDataType=sfix(16)  OutScaling=2^0
  Accumulator : xbsIndex_r4/Accumulator  operation=Add  n_bits=1  overflow=Saturate  scale=1  rst=on  hasbypass=off  en=off  dbl_ovrd=off  use_behavioral_HDL=on  implementation=Fabric  block_type=accum
      settings: infoedit="Adder or subtractor-based accumulator.   Output type and binary point position match the input.<P><P>Hardware notes: When \"Reinitialize with input 'b' on reset\" is selected, the accumulator is forced to run at the system rate even if the input 'b' is running at a slower rate."  xl_area=[0, 0, 0, 0, 0, 0, 0]  block_version=VER_STRING_GOES_HERE
  Accumulator2 : xbsIndex_r4/Accumulator  operation=Add  n_bits=14  overflow=Wrap  scale=1  rst=on  hasbypass=off  en=on  dbl_ovrd=off  use_behavioral_HDL=on  implementation=Fabric  block_type=accum
      settings: infoedit="Adder or subtractor-based accumulator.   Output type and binary point position match the input.<P><P>Hardware notes: When \"Reinitialize with input 'b' on reset\" is selected, the accumulator is forced to run at the system rate even if the input 'b' is running at a slower rate."  xl_area=[7 14 0 14 0 0 0]  block_version=VER_STRING_GOES_HERE
  AddSub1 : xbsIndex_r4/AddSub  Orientation=left  mode=Addition  use_carryin=off  use_carryout=off  en=off  latency=0  precision=Full  arith_type=Unsigned  n_bits=8  bin_pt=2  quantization=Truncate  overflow=Wrap  dbl_ovrd=off  use_behavioral_HDL=on  pipelined=off  use_rpm=on  hw_selection=Fabric  block_type=addsub
      settings: xl_area=[8 0 0 14 0 0 0]  block_version=VER_STRING_GOES_HERE
  Assert : xbsIndex_r4/Assert  Orientation=left  assert_type=off  type_source=Explicitly  arith_type="Signed  (2's comp)"  n_bits=16  bin_pt=14  assert_rate=on  rate_source=Explicitly  period=2  output_port=on  block_type=assert
      settings: infoedit=Asserts a user-defined sample rate and/or type on a signal.<P><P>Hardware notes: In hardware this block costs nothing.  block_version=VER_STRING_GOES_HERE
  Concat : xbsIndex_r4/Concat  num_inputs=3  dbl_ovrd=off  block_type=concat
      settings: block_version=VER_STRING_GOES_HERE
  Concat1 : Subsystem
  Concat2 : xbsIndex_r4/Concat  num_inputs=4  dbl_ovrd=off  block_type=concat
      settings: block_version=VER_STRING_GOES_HERE
  Constant2 : xbsIndex_r4/Constant  arith_type=Unsigned  const=0  n_bits=3  bin_pt=0  explicit_period=off  period=1  equ=P=C  opselect=C  inp2=PCIN>>17  opr=+  inp1=P  carry=CIN  dbl_ovrd=off  block_type=constant
      settings: block_version=VER_STRING_GOES_HERE
  Convert : xbsIndex_r4/Convert  arith_type=Unsigned  n_bits=1  bin_pt=0  quantization=Truncate  overflow=Wrap  latency=0  dbl_ovrd=off  pipeline=off  block_type=convert
      settings: xl_area=[0 0 0 0 0 0 0]  block_version=VER_STRING_GOES_HERE
  Convert1 : xbsIndex_r4/Convert  arith_type=Unsigned  n_bits=1  bin_pt=0  quantization=Truncate  overflow=Wrap  latency=0  dbl_ovrd=off  pipeline=off  block_type=convert
      settings: xl_area=[0 0 0 0 0 0 0]  block_version=VER_STRING_GOES_HERE
  Convert2 : xbsIndex_r4/Convert  Orientation=down  arith_type=Boolean  n_bits=1  bin_pt=0  quantization=Truncate  overflow=Wrap  latency=0  dbl_ovrd=off  pipeline=off  block_type=convert
      settings: xl_area=[0 0 0 0 0 0 0]  block_version=VER_STRING_GOES_HERE
  Convert3 : xbsIndex_r4/Convert  arith_type=Unsigned  n_bits=1  bin_pt=0  quantization=Truncate  overflow=Wrap  latency=0  dbl_ovrd=off  pipeline=off  block_type=convert
      settings: block_version=VER_STRING_GOES_HERE
  Delay1 : xbsIndex_r4/Delay  en=off  latency=2  dbl_ovrd=off  reg_retiming=off  block_type=delay
      settings: infoedit=Hardware notes: A delay line is a chain, each link of which is an SRL16 followed by a flip-flop. If register retiming is enabled, the delay line is a chain of flip-flops.  xl_area=[1 1 0 1 0 0 0]  block_version=VER_STRING_GOES_HERE
  Inverter : xbsIndex_r4/Inverter  en=off  latency=0  dbl_ovrd=off  block_type=inv
      settings: xl_area=[0 0 0 0 0 0 0]  block_version=VER_STRING_GOES_HERE
  Inverter1 : xbsIndex_r4/Inverter  en=off  latency=0  dbl_ovrd=off  block_type=inv
      settings: xl_area=[0 0 0 0 0 0 0]  block_version=VER_STRING_GOES_HERE
  Inverter2 : xbsIndex_r4/Inverter  Orientation=up  en=off  latency=0  dbl_ovrd=off  block_type=inv
      settings: xl_area=[0 0 0 0 0 0 0]  block_version=VER_STRING_GOES_HERE
  Inverter3 : xbsIndex_r4/Inverter  Orientation=down  en=off  latency=0  dbl_ovrd=off  block_type=inv
      settings: xl_area=[0 0 0 0 0 0 0]  block_version=VER_STRING_GOES_HERE
  Inverter4 : xbsIndex_r4/Inverter  en=off  latency=0  dbl_ovrd=off  block_type=inv
      settings: xl_area=[0 0 0 0 0 0 0]  block_version=VER_STRING_GOES_HERE
  Logical : xbsIndex_r4/Logical  logical_function=AND  inputs=2  en=off  latency=0  precision=Full  arith_type=Unsigned  n_bits=8  bin_pt=2  align_bp=on  dbl_ovrd=off  block_type=logical
      settings: xl_area=[4 0 0 4 0 0 0]  block_version=VER_STRING_GOES_HERE
  Logical1 : xbsIndex_r4/Logical  logical_function=AND  inputs=2  en=off  latency=0  precision=Full  arith_type=Unsigned  n_bits=8  bin_pt=2  align_bp=on  dbl_ovrd=off  block_type=logical
      settings: xl_area=[1 0 0 1 0 0 0]  block_version=VER_STRING_GOES_HERE
  Logical2 : xbsIndex_r4/Logical  logical_function=OR  inputs=3  en=off  latency=0  precision=Full  arith_type=Unsigned  n_bits=8  bin_pt=2  align_bp=on  dbl_ovrd=off  block_type=logical
      settings: xl_area=[1 0 0 1 0 0 0]  block_version=VER_STRING_GOES_HERE
  Logical3 : xbsIndex_r4/Logical  logical_function=AND  inputs=3  en=off  latency=0  precision=Full  arith_type=Unsigned  n_bits=8  bin_pt=2  align_bp=on  dbl_ovrd=off  block_type=logical
      settings: xl_area=[1 0 0 1 0 0 0]  block_version=VER_STRING_GOES_HERE
  Logical4 : xbsIndex_r4/Logical  logical_function=OR  inputs=2  en=off  latency=0  precision=Full  arith_type=Unsigned  n_bits=8  bin_pt=2  align_bp=on  dbl_ovrd=off  block_type=logical
      settings: xl_area=[1 0 0 1 0 0 0]  block_version=VER_STRING_GOES_HERE
  Register1 : xbsIndex_r4/Register  Orientation=left  init=0  rst=off  en=off  dbl_ovrd=off  block_type=register
      settings: xl_area=[8 15 0 0 0 0 0]  block_version=VER_STRING_GOES_HERE
  Register3 : xbsIndex_r4/Register  init=0  rst=off  en=off  dbl_ovrd=off  block_type=register
      settings: xl_area=[1 1 0 0 0 0 0]  block_version=VER_STRING_GOES_HERE
  Relational : xbsIndex_r4/Relational  mode=a<=b  en=off  latency=0  dbl_ovrd=off  block_type=relational
      settings: xl_area=[8 0 0 16 0 0 0]  block_version=VER_STRING_GOES_HERE
  Shift : xbsIndex_r4/Shift  shift_dir=Left  shift_bits=3  en=off  latency=0  precision=Full  arith_type="Signed  (2's comp)"  n_bits=8  bin_pt=2  quantization=Truncate  overflow=Wrap  dbl_ovrd=off  block_type=shift
      settings: xl_area=[0 0 0 0 0 0 0]  block_version=VER_STRING_GOES_HERE
  Tx Modulation : Subsystem
  ending : Subsystem
  mod-16 : xbsIndex_r4/Slice  nbits=4  boolean_output=off  mode=Lower Bit Location + Width  bit1=0  base1=MSB of Input  bit0=0  base0=LSB of Input  dbl_ovrd=off  block_type=slice
      settings: infoedit="Extracts a given range of bits from each input sample and presents it at the output.  The output type is ordinarily unsigned with binary point at zero, but can be Boolean when the slice is one bit wide.<P><P>Hardware notes: In hardware this block costs nothing."  block_version=VER_STRING_GOES_HERE
  off1 : xbsIndex_r4/Slice  nbits=1  boolean_output=on  mode=Lower Bit Location + Width  bit1=0  base1=MSB of Input  bit0=0  base0=LSB of Input  dbl_ovrd=off  block_type=slice
      settings: infoedit="Extracts a given range of bits from each input sample and presents it at the output.  The output type is ordinarily unsigned with binary point at zero, but can be Boolean when the slice is one bit wide.<P><P>Hardware notes: In hardware this block costs nothing."  block_version=VER_STRING_GOES_HERE
  on : xbsIndex_r4/Slice  nbits=1  boolean_output=on  mode=Lower Bit Location + Width  bit1=1  base1=MSB of Input  bit0=2  base0=LSB of Input  dbl_ovrd=off  block_type=slice
      settings: infoedit="Extracts a given range of bits from each input sample and presents it at the output.  The output type is ordinarily unsigned with binary point at zero, but can be Boolean when the slice is one bit wide.<P><P>Hardware notes: In hardware this block costs nothing."  block_version=VER_STRING_GOES_HERE
  on_resetting_off1 : xbsIndex_r4/Register  init=1  rst=on  en=on  dbl_ovrd=off  block_type=register
      settings: xl_area=[2 3 0 0 0 0 0]  block_version=VER_STRING_GOES_HERE
  reset1 : xbsIndex_r4/Logical  logical_function=AND  inputs=2  en=off  latency=0  precision=Full  arith_type=Unsigned  n_bits=8  bin_pt=2  align_bp=on  dbl_ovrd=off  block_type=logical
      settings: xl_area=[1 0 0 1 0 0 0]  block_version=VER_STRING_GOES_HERE
  reset2 : xbsIndex_r4/Slice  nbits=1  boolean_output=on  mode=Lower Bit Location + Width  bit1=1  base1=MSB of Input  bit0=1  base0=LSB of Input  dbl_ovrd=off  block_type=slice
      settings: infoedit="Extracts a given range of bits from each input sample and presents it at the output.  The output type is ordinarily unsigned with binary point at zero, but can be Boolean when the slice is one bit wide.<P><P>Hardware notes: In hardware this block costs nothing."  block_version=VER_STRING_GOES_HERE
  reset3 : xbsIndex_r4/Logical  logical_function=AND  inputs=2  en=off  latency=0  precision=Full  arith_type=Unsigned  n_bits=8  bin_pt=2  align_bp=on  dbl_ovrd=off  block_type=logical
      settings: xl_area=[1 0 0 1 0 0 0]  block_version=VER_STRING_GOES_HERE
  reset : Outport  OutDataType=sfix(16)  OutScaling=2^0
  index : Outport  Port=2  OutDataType=sfix(16)  OutScaling=2^0
  mod : Outport  Port=3  OutDataType=sfix(16)  OutScaling=2^0
  wires:
  Register1/1 -> Assert/1
  on/1 -> Logical3/2
  Logical3/1 -> Convert/1
  Concat2/1 -> Logical/1
  Convert/1 -> Concat2/4, Concat2/3, Concat2/2, Concat2/1
  valid/1 -> Register3/1, Inverter2/1
  Logical/1 -> AddSub1/1, Accumulator2/1, mod/1
  Inverter1/1 -> Accumulator2/3
  reset2/1 -> Accumulator2/2
  Concat/1 -> on_resetting_off1/1
  Logical2/1 -> on_resetting_off1/3
  Logical1/1 -> Logical2/1, Concat/3
  Delay1/1 -> Logical2/3, Concat/1
  reset1/1 -> Concat/2, reset/1, Logical2/2, Delay1/1
  Logical4/1 -> reset1/2
  off1/1 -> Inverter1/1, Logical4/2
  Inverter/1 -> Logical1/2
  ending/1(pulse) -> Logical4/1, Logical1/1, Convert1/1
  AddSub1/1 -> Register1/1
  mod-16/1 -> index/1
  Constant2/1 -> Concat1/1(in0)
  Concat1/1(out0) -> Shift/1
  length/1 -> Concat1/2(in1)
  Accumulator2/1 -> mod-16/1, AddSub1/2
  on_resetting_off1/1 -> on/1, reset2/1, off1/1
  Relational/1 -> ending/1(signal)
  Shift/1 -> Relational/1
  Register3/1 -> Logical3/3
  Inverter2/1 -> Accumulator/2
  Accumulator/1 -> Inverter3/1
  Convert1/1 -> Accumulator/1
  Convert2/1 -> Logical3/1
  Inverter3/1 -> Convert2/1
  Tx Modulation/1(mod) -> Logical/2
  reset3/1 -> reset1/1, Inverter/1
  [TxReset] -> on_resetting_off1/2
  ready/1 -> reset3/1
  [TxReset] -> Inverter4/1
  Inverter4/1 -> reset3/2
  BaseRate_En/1 -> Convert3/1
  Convert3/1 -> Tx Modulation/1(BaseRate)
  ifft_index/1 -> Tx Modulation/3(IFFT Index)
  ant_sel/1 -> Tx Modulation/2(Ant Sel)
  Assert/1 -> Relational/2
  FullRateMasks/1 -> Tx Modulation/4(FullRateMasks)
